simulink model "HvacCtrlrAirTFrnt"  (R2022b)
settings: Solver=FixedStepDiscrete  StartTime=0.0  StopTime=10.0  FixedStep=0.2  RelTol=1e-3
settings Model: Creator=blue.ge  LastModifiedBy=blue.ge
settings DataIOCC: SaveFormat=StructureWithTime
settings OptimizationCC: DefaultParameterBehavior=Inlined  EnhancedBackFolding=on  PassReuseOutputArgsAs=Individual arguments  ZeroExternalMemoryAtStartup=off  ZeroInternalMemoryAtStartup=off  OptimizeBlockOrder=speed  DifferentSizesBufferReuse=on  OptimizationCustomize=off  EfficientTunableParamExpr=on
settings DebuggingCC: ParameterTunabilityLossMsg=error
settings ModelReferenceCC: ModelReferenceNumInstancesAllowed=Single
settings SFSimCC: MATLABDynamicMemAlloc=off
settings RTWCC: Description=AUTOSAR  SystemTargetFile=autosar.tlc  GenCodeOnly=on  TemplateMakefile=ert_default_tmf
settings CodeAppCC: ForceParamTrailComments=on  IgnoreCustomStorageClasses=off  OperatorAnnotations=on  SimulinkDataObjDesc=on  SFDataObjDesc=on  InsertBlockDesc=on
settings STFCustomTargetCC: TargetLangStandard=C99 (ISO)  MultiwordLength=256  ParMdlRefBuildCompliant=on  CompOptLevelCompliant=on  IncludeMdlTerminateFcn=off  CombineOutputUpdateFcns=on  SuppressErrorStatus=on  ERTCustomFileBanners=on  SupportNonFinite=off  SupportComplex=off  SupportContinuousTime=off  SupportNonInlinedSFcns=off  RemoveResetFunc=on  MATLABClassNameForMDSCustomization=Simulink.SoftwareTarget.ERTCustomization  AutosarCompliant=on  UseToolchainInfoCompliant=on  LookupTableObjectStructAxisOrder=2,1,3,4,...  LUTObjectStructOrderEvenSpacing=Size,Table,Breakpoints  SystemTargetFile=autosar.tlc
settings ERTTargetCC: TargetLangStandard=C99 (ISO)  ConcurrentExecutionCompliant=off  IncludeMdlTerminateFcn=off  SuppressErrorStatus=on  SupportNonFinite=off  SupportComplex=off  ModelStepFunctionPrototypeControlCompliant=off  CPPClassGenCompliant=off  AutosarCompliant=on  LookupTableObjectStructAxisOrder=2,1,3,4,...  LUTObjectStructOrderEvenSpacing=Size,Table,Breakpoints  InlinedParameterPlacement=NonHierarchical  ERTCustomFileTemplate=example_file_process.tlc

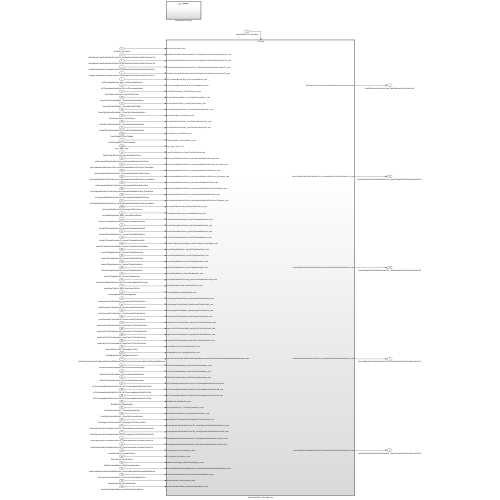
[thumbnail: Simulink's stored preview of the top-level diagram]
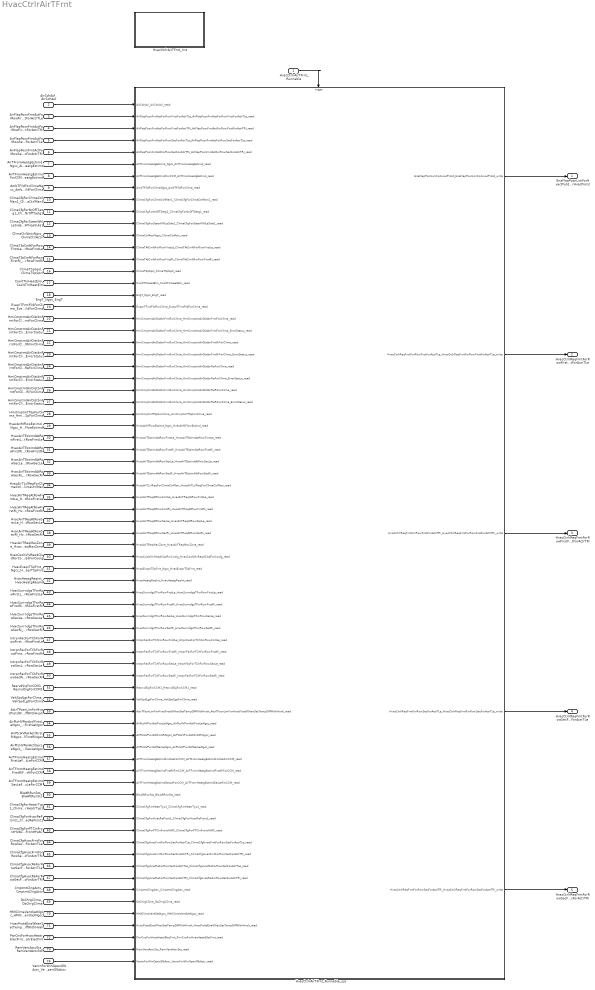
== system HvacCtrlrAirTFrnt ==
  HvacCtrlrAirTFrnt_Runnable : Inport  SampleTime=0.2  OutputFunctionCall=on
  AirCdnAvl_AirCdnAvl : Inport  Port=2  OutDataTypeStr=Boolean  PortDimensions=1
  AirFlapPosnFrntActForRowFirstForActrTLe_AirFlapPosnFrntActForRowFirstForActrTLe : Inport  Port=3  OutMin=0  OutMax=100  OutDataTypeStr=Perc9  PortDimensions=1
  AirFlapPosnFrntActForRowFirstForActrTRi_AirFlapPosnFrntActForRowFirstForActrTRi : Inport  Port=4  OutMin=0  OutMax=100  OutDataTypeStr=Perc9  PortDimensions=1
  AirFlapPosnFrntActForRowSecForActrTLe_AirFlapPosnFrntActForRowSecForActrTLe : Inport  Port=5  OutMin=0  OutMax=100  OutDataTypeStr=Perc9  PortDimensions=1
  AirFlapPosnFrntActForRowSecForActrTRi_AirFlapPosnFrntActForRowSecForActrTRi : Inport  Port=6  OutMin=0  OutMax=100  OutDataTypeStr=Perc9  PortDimensions=1
  AirTFromHeatgEstimd_Ngcc_AirTFromHeatgEstimd : Inport  Port=7  OutDataTypeStr=T30  PortDimensions=1
  AirTFromHeatgEstimdForCCM_AirTFromHeatgEstimd : Inport  Port=8  OutDataTypeStr=T30  PortDimensions=1
  AmbTFildForClimaNgcc_AmbTFildForClima : Inport  Port=9  OutDataTypeStr=T30  PortDimensions=1
  ClimaCfgForClimaCtrlMan1_ClimaCfgForClimaCtrlMan1 : Inport  Port=10  OutDataTypeStr=Boolean  PortDimensions=1
  ClimaCfgForNrOfTSetg1_ClimaCfgForNrOfTSetg1 : Inport  Port=11  OutDataTypeStr=Enum: ClimaCfgForNrOfTSetg1  PortDimensions=1
  ClimaCfgForSteerWhlLeSide1_ClimaCfgForSteerWhlLeSide1 : Inport  Port=12  OutDataTypeStr=Boolean  PortDimensions=1
  ClimaCtrlActvNgcc_ClimaCtrlActv : Inport  Port=13  OutDataTypeStr=Boolean  PortDimensions=1
  ClimaTAtCmftForRowFirstLe_ClimaTAtCmftForRowFirstLe : Inport  Port=14  OutDataTypeStr=T30  PortDimensions=1
  ClimaTAtCmftForRowFirstRi_ClimaTAtCmftForRowFirstRi : Inport  Port=15  OutDataTypeStr=T30  PortDimensions=1
  ClimaTSpSpcl_ClimaTSpSpcl : Inport  Port=16  OutDataTypeStr=Bus: ClimaTSpSpcl  PortDimensions=1  BusOutputAsStruct=on
  CooltTInHeatElm_CooltTInHeatElm : Inport  Port=17  OutDataTypeStr=T30  PortDimensions=1
  EngT_Ngcc_EngT : Inport  Port=18  OutDataTypeStr=Bus: EngT  PortDimensions=1  BusOutputAsStruct=on
  EvaprTFrntFildForClima_EvaprTFrntFildForClima : Inport  Port=19  OutDataTypeStr=T30  PortDimensions=1
  HmiCmptmtAirDistbnFrntForClima_HmiCmptmtAirDistbnFrntForClima : Inport  Port=20  OutDataTypeStr=Enum: HmiCmptmtAirDistbnFrnt  PortDimensions=1
  HmiCmptmtAirDistbnFrntForClima_HmiCmptmtAirDistbnFrntForClima_ErrorStatus : Inport  Port=21  OutDataTypeStr=uint8  PortDimensions=1
  HmiCmptmtAirDistbnFrntForClima_HmiCmptmtAirDistbnFrntRiForClima : Inport  Port=22  OutDataTypeStr=Enum: HmiCmptmtAirDistbnFrnt  PortDimensions=1
  HmiCmptmtAirDistbnFrntForClima_HmiCmptmtAirDistbnFrntRiForClima_ErrorStatus : Inport  Port=23  OutDataTypeStr=uint8  PortDimensions=1
  HmiCmptmtAirDistbnFrntForClima_HmiCmptmtAirDistbnReForClima : Inport  Port=24  OutDataTypeStr=Enum: HmiCmptmtAirDistbnFrnt  PortDimensions=1
  HmiCmptmtAirDistbnFrntForClima_HmiCmptmtAirDistbnReForClima_ErrorStatus : Inport  Port=25  OutDataTypeStr=uint8  PortDimensions=1
  HmiCmptmtAirDistbnFrntForClima_HmiCmptmtAirDistbnReRiForClima : Inport  Port=26  OutDataTypeStr=Enum: HmiCmptmtAirDistbnFrnt  PortDimensions=1
  HmiCmptmtAirDistbnFrntForClima_HmiCmptmtAirDistbnReRiForClima_ErrorStatus : Inport  Port=27  OutDataTypeStr=uint8  PortDimensions=1
  HmiCmptmtTSpForClima_HmiCmptmtTSpForClima : Inport  Port=28  OutDataTypeStr=Bus: HmiCmptmtTSp  PortDimensions=1  BusOutputAsStruct=on
  HvacAirMFlowEstimd_Ngcc_HvacAirMFlowEstimd : Inport  Port=29  OutMin=0  OutMax=1000  OutDataTypeStr=MFlow1  PortDimensions=1
  HvacAirTEstimdAtRowFirstLe_HvacAirTEstimdAtRowFirstLe : Inport  Port=30  OutDataTypeStr=T30  PortDimensions=1
  HvacAirTEstimdAtRowFirstRi_HvacAirTEstimdAtRowFirstRi : Inport  Port=31  OutDataTypeStr=T30  PortDimensions=1
  HvacAirTEstimdAtRowSecLe_HvacAirTEstimdAtRowSecLe : Inport  Port=32  OutDataTypeStr=T30  PortDimensions=1
  HvacAirTEstimdAtRowSecRi_HvacAirTEstimdAtRowSecRi : Inport  Port=33  OutDataTypeStr=T30  PortDimensions=1
  HvacAirTLvlReqForClimaCtrlMan_HvacAirTLvlReqForClimaCtrlMan : Inport  Port=34  OutDataTypeStr=Perc30  PortDimensions=1
  HvacAirTReqAtRowFirstLe_HvacAirTReqAtRowFirstLe : Inport  Port=35  OutDataTypeStr=T30  PortDimensions=1
  HvacAirTReqAtRowFirstRi_HvacAirTReqAtRowFirstRi : Inport  Port=36  OutDataTypeStr=T30  PortDimensions=1
  HvacAirTReqAtRowSecLe_HvacAirTReqAtRowSecLe : Inport  Port=37  OutDataTypeStr=T30  PortDimensions=1
  HvacAirTReqAtRowSecRi_HvacAirTReqAtRowSecRi : Inport  Port=38  OutDataTypeStr=T30  PortDimensions=1
  HvacAirTReqMaxZone_HvacAirTReqMaxZone : Inport  Port=39  OutDataTypeStr=Enum: TempZone  PortDimensions=1
  HvacCooltVlvReqdClsdForCoolg_HvacCooltVlvReqdClsdForCoolg : Inport  Port=40  OutDataTypeStr=Boolean  PortDimensions=1
  HvacEvaprTSpFrnt_Ngcc_HvacEvaprTSpFrnt : Inport  Port=41  OutDataTypeStr=HvacEvaprTSp  PortDimensions=1
  HvacHeatgReqInt_HvacHeatgReqInt : Inport  Port=42  OutDataTypeStr=Enum: OnOff1  PortDimensions=1
  HvacSurrndgsTForRowFirstLe_HvacSurrndgsTForRowFirstLe : Inport  Port=43  OutDataTypeStr=T30  PortDimensions=1
  HvacSurrndgsTForRowFirstRi_HvacSurrndgsTForRowFirstRi : Inport  Port=44  OutDataTypeStr=T30  PortDimensions=1
  HvacSurrndgsTForRowSecLe_HvacSurrndgsTForRowSecLe : Inport  Port=45  OutDataTypeStr=T30  PortDimensions=1
  HvacSurrndgsTForRowSecRi_HvacSurrndgsTForRowSecRi : Inport  Port=46  OutDataTypeStr=T30  PortDimensions=1
  IntrpnFacForTChForRowFirstLe_IntrpnFacForTChForRowFirstLe : Inport  Port=47  OutDataTypeStr=Fac30  PortDimensions=1
  IntrpnFacForTChForRowFirstRi_IntrpnFacForTChForRowFirstRi : Inport  Port=48  OutDataTypeStr=Fac30  PortDimensions=1
  IntrpnFacForTChForRowSecLe_IntrpnFacForTChForRowSecLe : Inport  Port=49  OutDataTypeStr=Fac30  PortDimensions=1
  IntrpnFacForTChForRowSecRi_IntrpnFacForTChForRowSecRi : Inport  Port=50  OutDataTypeStr=Fac30  PortDimensions=1
  ResrvdSigForCCM1_ResrvdSigForCCM1 : Inport  Port=51  OutDataTypeStr=BattIdTbl  PortDimensions=1
  VehSpdLgtForClima_VehSpdLgtForClima : Inport  Port=52  OutDataTypeStr=Bus: VehSpdLgtForClima  PortDimensions=1  BusOutputAsStruct=on
  ActrTPosnLimForHvacProtdWhenSetTempDiffWithHvah_ActrTPosnLimForHvacProtdWhenSetTempDiffWithHvah : Inport  Port=53  OutDataTypeStr=VCCPerc30  PortDimensions=1
  AirPtcHVPwrActlFirstLeNgcc_AirPtcHVPwrActlFirstLeNgcc : Inport  Port=54  OutMin=0  OutMax=20460  OutDataTypeStr=Pwr3  PortDimensions=1
  AirPtcHVPwrActlFirstRiNgcc_AirPtcHVPwrActlFirstRiNgcc : Inport  Port=55  OutMin=0  OutMax=20460  OutDataTypeStr=Pwr3  PortDimensions=1
  AirPtcHVPwrActlSecLeNgcc_AirPtcHVPwrActlSecLeNgcc : Inport  Port=56  OutMin=0  OutMax=20460  OutDataTypeStr=Pwr3  PortDimensions=1
  AirTFromHeatgEstimdFirstLeForCCM_AirTFromHeatgEstimdFirstLeForCCM : Inport  Port=57  OutDataTypeStr=T30  PortDimensions=1
  AirTFromHeatgEstimdFirstRiForCCM_AirTFromHeatgEstimdFirstRiForCCM : Inport  Port=58  OutDataTypeStr=T30  PortDimensions=1
  AirTFromHeatgEstimdSecLeForCCM_AirTFromHeatgEstimdSecLeForCCM : Inport  Port=59  OutDataTypeStr=T30  PortDimensions=1
  BlwAftRunSts_BlwAftRunSts : Inport  Port=60  OutDataTypeStr=Boolean  PortDimensions=1
  ClimaCfgForHeatrTyp1_ClimaCfgForHeatrTyp1 : Inport  Port=61  OutDataTypeStr=Enum: ClimaCfgForHeatrTyp1  PortDimensions=1
  ClimaCfgForHvacRePrsnt1_ClimaCfgForHvacRePrsnt1 : Inport  Port=62  OutDataTypeStr=Enum: CarCfgForHvacRe  PortDimensions=1
  ClimaCfgForPTCInFrontHVAC_ClimaCfgForPTCInFrontHVAC : Inport  Port=63  OutDataTypeStr=Enum: FrontHeaterExchanger  PortDimensions=1
  ClimaCfgHvacFrntForRowSecForActrTLe_ClimaCfgHvacFrntForRowSecForActrTLe : Inport  Port=64  OutDataTypeStr=Boolean  PortDimensions=1
  ClimaCfgHvacFrntForRowSecForActrTRi_ClimaCfgHvacFrntForRowSecForActrTRi : Inport  Port=65  OutDataTypeStr=Boolean  PortDimensions=1
  ClimaCfgHvacReForRowSecForActrTLe_ClimaCfgHvacReForRowSecForActrTLe : Inport  Port=66  OutDataTypeStr=UInt8  PortDimensions=1
  ClimaCfgHvacReForRowSecForActrTRi_ClimaCfgHvacReForRowSecForActrTRi : Inport  Port=67  OutDataTypeStr=UInt8  PortDimensions=1
  CmptmtClngActv_CmptmtClngActv : Inport  Port=68  OutDataTypeStr=Enum: CmptmtClngActv  PortDimensions=1
  DsChrgClima_DsChrgClima : Inport  Port=69  OutDataTypeStr=Boolean  PortDimensions=1
  HMIClimaVentSetNgcc_HMIClimaVentSetNgcc : Inport  Port=70  OutDataTypeStr=Enum: OnOff1  PortDimensions=1
  HvacProtdEnaWhenSetTempDiffWithHvah_HvacProtdEnaWhenSetTempDiffWithHvah : Inport  Port=71  OutDataTypeStr=Boolean  PortDimensions=1
  PwrCnsForHvacHeatrElecFrnt_PwrCnsForHvacHeatrElecFrnt : Inport  Port=72  OutDataTypeStr=Pwr33  PortDimensions=1
  RemVentActvSts_RemVentActvSts : Inport  Port=73  OutDataTypeStr=Enum: OnOff1  PortDimensions=1
  VentnForWinOpenGlbActv_VentnForWinOpenGlbActv : Inport  Port=74  OutDataTypeStr=Boolean  PortDimensions=1
  HvacCtrlrAirTFrnt_Init : Subsystem
  HvacCtrlrAirTFrnt_Runnable_sys : Subsystem
  EnaFlapPosnLimForHvacProtd_EnaFlapPosnLimForHvacProtd : Outport  OutDataTypeStr=Boolean  PortDimensions=1
  HvacCtrlrReqFrntForRowFirstForActrTLe_HvacCtrlrReqFrntForRowFirstForActrTLe : Outport  Port=2  OutDataTypeStr=Perc30  PortDimensions=1
  HvacCtrlrReqFrntForRowFirstForActrTRi_HvacCtrlrReqFrntForRowFirstForActrTRi : Outport  Port=3  OutDataTypeStr=Perc30  PortDimensions=1
  HvacCtrlrReqFrntForRowSecForActrTLe_HvacCtrlrReqFrntForRowSecForActrTLe : Outport  Port=4  OutDataTypeStr=Perc30  PortDimensions=1
  HvacCtrlrReqFrntForRowSecForActrTRi_HvacCtrlrReqFrntForRowSecForActrTRi : Outport  Port=5  OutDataTypeStr=Perc30  PortDimensions=1
  wires:
  AirCdnAvl_AirCdnAvl/1 -> HvacCtrlrAirTFrnt_Runnable_sys/1(AirCdnAvl_AirCdnAvl_read)
  AirFlapPosnFrntActForRowFirstForActrTLe_AirFlapPosnFrntActForRowFirstForActrTLe/1 -> HvacCtrlrAirTFrnt_Runnable_sys/2(AirFlapPosnFrntActForRowFirstForActrTLe_AirFlapPosnFrntActForRowFirstForActrTLe_read)
  AirFlapPosnFrntActForRowFirstForActrTRi_AirFlapPosnFrntActForRowFirstForActrTRi/1 -> HvacCtrlrAirTFrnt_Runnable_sys/3(AirFlapPosnFrntActForRowFirstForActrTRi_AirFlapPosnFrntActForRowFirstForActrTRi_read)
  AirFlapPosnFrntActForRowSecForActrTLe_AirFlapPosnFrntActForRowSecForActrTLe/1 -> HvacCtrlrAirTFrnt_Runnable_sys/4(AirFlapPosnFrntActForRowSecForActrTLe_AirFlapPosnFrntActForRowSecForActrTLe_read)
  AirFlapPosnFrntActForRowSecForActrTRi_AirFlapPosnFrntActForRowSecForActrTRi/1 -> HvacCtrlrAirTFrnt_Runnable_sys/5(AirFlapPosnFrntActForRowSecForActrTRi_AirFlapPosnFrntActForRowSecForActrTRi_read)
  AirTFromHeatgEstimd_Ngcc_AirTFromHeatgEstimd/1 -> HvacCtrlrAirTFrnt_Runnable_sys/6(AirTFromHeatgEstimd_Ngcc_AirTFromHeatgEstimd_read)
  AirTFromHeatgEstimdForCCM_AirTFromHeatgEstimd/1 -> HvacCtrlrAirTFrnt_Runnable_sys/7(AirTFromHeatgEstimdForCCM_AirTFromHeatgEstimd_read)
  AmbTFildForClimaNgcc_AmbTFildForClima/1 -> HvacCtrlrAirTFrnt_Runnable_sys/8(AmbTFildForClimaNgcc_AmbTFildForClima_read)
  ClimaCfgForClimaCtrlMan1_ClimaCfgForClimaCtrlMan1/1 -> HvacCtrlrAirTFrnt_Runnable_sys/9(ClimaCfgForClimaCtrlMan1_ClimaCfgForClimaCtrlMan1_read)
  ClimaCfgForNrOfTSetg1_ClimaCfgForNrOfTSetg1/1 -> HvacCtrlrAirTFrnt_Runnable_sys/10(ClimaCfgForNrOfTSetg1_ClimaCfgForNrOfTSetg1_read)
  ClimaCfgForSteerWhlLeSide1_ClimaCfgForSteerWhlLeSide1/1 -> HvacCtrlrAirTFrnt_Runnable_sys/11(ClimaCfgForSteerWhlLeSide1_ClimaCfgForSteerWhlLeSide1_read)
  ClimaCtrlActvNgcc_ClimaCtrlActv/1 -> HvacCtrlrAirTFrnt_Runnable_sys/12(ClimaCtrlActvNgcc_ClimaCtrlActv_read)
  ClimaTAtCmftForRowFirstLe_ClimaTAtCmftForRowFirstLe/1 -> HvacCtrlrAirTFrnt_Runnable_sys/13(ClimaTAtCmftForRowFirstLe_ClimaTAtCmftForRowFirstLe_read)
  ClimaTAtCmftForRowFirstRi_ClimaTAtCmftForRowFirstRi/1 -> HvacCtrlrAirTFrnt_Runnable_sys/14(ClimaTAtCmftForRowFirstRi_ClimaTAtCmftForRowFirstRi_read)
  ClimaTSpSpcl_ClimaTSpSpcl/1 -> HvacCtrlrAirTFrnt_Runnable_sys/15(ClimaTSpSpcl_ClimaTSpSpcl_read)
  CooltTInHeatElm_CooltTInHeatElm/1 -> HvacCtrlrAirTFrnt_Runnable_sys/16(CooltTInHeatElm_CooltTInHeatElm_read)
  EngT_Ngcc_EngT/1 -> HvacCtrlrAirTFrnt_Runnable_sys/17(EngT_Ngcc_EngT_read)
  EvaprTFrntFildForClima_EvaprTFrntFildForClima/1 -> HvacCtrlrAirTFrnt_Runnable_sys/18(EvaprTFrntFildForClima_EvaprTFrntFildForClima_read)
  HmiCmptmtAirDistbnFrntForClima_HmiCmptmtAirDistbnFrntForClima/1 -> HvacCtrlrAirTFrnt_Runnable_sys/19(HmiCmptmtAirDistbnFrntForClima_HmiCmptmtAirDistbnFrntForClima_read)
  HmiCmptmtAirDistbnFrntForClima_HmiCmptmtAirDistbnFrntForClima_ErrorStatus/1 -> HvacCtrlrAirTFrnt_Runnable_sys/20(HmiCmptmtAirDistbnFrntForClima_HmiCmptmtAirDistbnFrntForClima_ErrorStatus_read)
  HmiCmptmtAirDistbnFrntForClima_HmiCmptmtAirDistbnFrntRiForClima/1 -> HvacCtrlrAirTFrnt_Runnable_sys/21(HmiCmptmtAirDistbnFrntForClima_HmiCmptmtAirDistbnFrntRiForClima_read)
  HmiCmptmtAirDistbnFrntForClima_HmiCmptmtAirDistbnFrntRiForClima_ErrorStatus/1 -> HvacCtrlrAirTFrnt_Runnable_sys/22(HmiCmptmtAirDistbnFrntForClima_HmiCmptmtAirDistbnFrntRiForClima_ErrorStatus_read)
  HmiCmptmtAirDistbnFrntForClima_HmiCmptmtAirDistbnReForClima/1 -> HvacCtrlrAirTFrnt_Runnable_sys/23(HmiCmptmtAirDistbnFrntForClima_HmiCmptmtAirDistbnReForClima_read)
  HmiCmptmtAirDistbnFrntForClima_HmiCmptmtAirDistbnReForClima_ErrorStatus/1 -> HvacCtrlrAirTFrnt_Runnable_sys/24(HmiCmptmtAirDistbnFrntForClima_HmiCmptmtAirDistbnReForClima_ErrorStatus_read)
  HmiCmptmtAirDistbnFrntForClima_HmiCmptmtAirDistbnReRiForClima/1 -> HvacCtrlrAirTFrnt_Runnable_sys/25(HmiCmptmtAirDistbnFrntForClima_HmiCmptmtAirDistbnReRiForClima_read)
  HmiCmptmtAirDistbnFrntForClima_HmiCmptmtAirDistbnReRiForClima_ErrorStatus/1 -> HvacCtrlrAirTFrnt_Runnable_sys/26(HmiCmptmtAirDistbnFrntForClima_HmiCmptmtAirDistbnReRiForClima_ErrorStatus_read)
  HmiCmptmtTSpForClima_HmiCmptmtTSpForClima/1 -> HvacCtrlrAirTFrnt_Runnable_sys/27(HmiCmptmtTSpForClima_HmiCmptmtTSpForClima_read)
  HvacAirMFlowEstimd_Ngcc_HvacAirMFlowEstimd/1 -> HvacCtrlrAirTFrnt_Runnable_sys/28(HvacAirMFlowEstimd_Ngcc_HvacAirMFlowEstimd_read)
  HvacAirTEstimdAtRowFirstLe_HvacAirTEstimdAtRowFirstLe/1 -> HvacCtrlrAirTFrnt_Runnable_sys/29(HvacAirTEstimdAtRowFirstLe_HvacAirTEstimdAtRowFirstLe_read)
  HvacAirTEstimdAtRowFirstRi_HvacAirTEstimdAtRowFirstRi/1 -> HvacCtrlrAirTFrnt_Runnable_sys/30(HvacAirTEstimdAtRowFirstRi_HvacAirTEstimdAtRowFirstRi_read)
  HvacAirTEstimdAtRowSecLe_HvacAirTEstimdAtRowSecLe/1 -> HvacCtrlrAirTFrnt_Runnable_sys/31(HvacAirTEstimdAtRowSecLe_HvacAirTEstimdAtRowSecLe_read)
  HvacAirTEstimdAtRowSecRi_HvacAirTEstimdAtRowSecRi/1 -> HvacCtrlrAirTFrnt_Runnable_sys/32(HvacAirTEstimdAtRowSecRi_HvacAirTEstimdAtRowSecRi_read)
  HvacAirTLvlReqForClimaCtrlMan_HvacAirTLvlReqForClimaCtrlMan/1 -> HvacCtrlrAirTFrnt_Runnable_sys/33(HvacAirTLvlReqForClimaCtrlMan_HvacAirTLvlReqForClimaCtrlMan_read)
  HvacAirTReqAtRowFirstLe_HvacAirTReqAtRowFirstLe/1 -> HvacCtrlrAirTFrnt_Runnable_sys/34(HvacAirTReqAtRowFirstLe_HvacAirTReqAtRowFirstLe_read)
  HvacAirTReqAtRowFirstRi_HvacAirTReqAtRowFirstRi/1 -> HvacCtrlrAirTFrnt_Runnable_sys/35(HvacAirTReqAtRowFirstRi_HvacAirTReqAtRowFirstRi_read)
  HvacAirTReqAtRowSecLe_HvacAirTReqAtRowSecLe/1 -> HvacCtrlrAirTFrnt_Runnable_sys/36(HvacAirTReqAtRowSecLe_HvacAirTReqAtRowSecLe_read)
  HvacAirTReqAtRowSecRi_HvacAirTReqAtRowSecRi/1 -> HvacCtrlrAirTFrnt_Runnable_sys/37(HvacAirTReqAtRowSecRi_HvacAirTReqAtRowSecRi_read)
  HvacAirTReqMaxZone_HvacAirTReqMaxZone/1 -> HvacCtrlrAirTFrnt_Runnable_sys/38(HvacAirTReqMaxZone_HvacAirTReqMaxZone_read)
  HvacCooltVlvReqdClsdForCoolg_HvacCooltVlvReqdClsdForCoolg/1 -> HvacCtrlrAirTFrnt_Runnable_sys/39(HvacCooltVlvReqdClsdForCoolg_HvacCooltVlvReqdClsdForCoolg_read)
  HvacEvaprTSpFrnt_Ngcc_HvacEvaprTSpFrnt/1 -> HvacCtrlrAirTFrnt_Runnable_sys/40(HvacEvaprTSpFrnt_Ngcc_HvacEvaprTSpFrnt_read)
  HvacHeatgReqInt_HvacHeatgReqInt/1 -> HvacCtrlrAirTFrnt_Runnable_sys/41(HvacHeatgReqInt_HvacHeatgReqInt_read)
  HvacSurrndgsTForRowFirstLe_HvacSurrndgsTForRowFirstLe/1 -> HvacCtrlrAirTFrnt_Runnable_sys/42(HvacSurrndgsTForRowFirstLe_HvacSurrndgsTForRowFirstLe_read)
  HvacSurrndgsTForRowFirstRi_HvacSurrndgsTForRowFirstRi/1 -> HvacCtrlrAirTFrnt_Runnable_sys/43(HvacSurrndgsTForRowFirstRi_HvacSurrndgsTForRowFirstRi_read)
  HvacSurrndgsTForRowSecLe_HvacSurrndgsTForRowSecLe/1 -> HvacCtrlrAirTFrnt_Runnable_sys/44(HvacSurrndgsTForRowSecLe_HvacSurrndgsTForRowSecLe_read)
  HvacSurrndgsTForRowSecRi_HvacSurrndgsTForRowSecRi/1 -> HvacCtrlrAirTFrnt_Runnable_sys/45(HvacSurrndgsTForRowSecRi_HvacSurrndgsTForRowSecRi_read)
  IntrpnFacForTChForRowFirstLe_IntrpnFacForTChForRowFirstLe/1 -> HvacCtrlrAirTFrnt_Runnable_sys/46(IntrpnFacForTChForRowFirstLe_IntrpnFacForTChForRowFirstLe_read)
  IntrpnFacForTChForRowFirstRi_IntrpnFacForTChForRowFirstRi/1 -> HvacCtrlrAirTFrnt_Runnable_sys/47(IntrpnFacForTChForRowFirstRi_IntrpnFacForTChForRowFirstRi_read)
  IntrpnFacForTChForRowSecLe_IntrpnFacForTChForRowSecLe/1 -> HvacCtrlrAirTFrnt_Runnable_sys/48(IntrpnFacForTChForRowSecLe_IntrpnFacForTChForRowSecLe_read)
  IntrpnFacForTChForRowSecRi_IntrpnFacForTChForRowSecRi/1 -> HvacCtrlrAirTFrnt_Runnable_sys/49(IntrpnFacForTChForRowSecRi_IntrpnFacForTChForRowSecRi_read)
  ResrvdSigForCCM1_ResrvdSigForCCM1/1 -> HvacCtrlrAirTFrnt_Runnable_sys/50(ResrvdSigForCCM1_ResrvdSigForCCM1_read)
  VehSpdLgtForClima_VehSpdLgtForClima/1 -> HvacCtrlrAirTFrnt_Runnable_sys/51(VehSpdLgtForClima_VehSpdLgtForClima_read)
  ActrTPosnLimForHvacProtdWhenSetTempDiffWithHvah_ActrTPosnLimForHvacProtdWhenSetTempDiffWithHvah/1 -> HvacCtrlrAirTFrnt_Runnable_sys/52(ActrTPosnLimForHvacProtdWhenSetTempDiffWithHvah_ActrTPosnLimForHvacProtdWhenSetTempDiffWithHvah_read)
  AirPtcHVPwrActlFirstLeNgcc_AirPtcHVPwrActlFirstLeNgcc/1 -> HvacCtrlrAirTFrnt_Runnable_sys/53(AirPtcHVPwrActlFirstLeNgcc_AirPtcHVPwrActlFirstLeNgcc_read)
  AirPtcHVPwrActlFirstRiNgcc_AirPtcHVPwrActlFirstRiNgcc/1 -> HvacCtrlrAirTFrnt_Runnable_sys/54(AirPtcHVPwrActlFirstRiNgcc_AirPtcHVPwrActlFirstRiNgcc_read)
  AirPtcHVPwrActlSecLeNgcc_AirPtcHVPwrActlSecLeNgcc/1 -> HvacCtrlrAirTFrnt_Runnable_sys/55(AirPtcHVPwrActlSecLeNgcc_AirPtcHVPwrActlSecLeNgcc_read)
  AirTFromHeatgEstimdFirstLeForCCM_AirTFromHeatgEstimdFirstLeForCCM/1 -> HvacCtrlrAirTFrnt_Runnable_sys/56(AirTFromHeatgEstimdFirstLeForCCM_AirTFromHeatgEstimdFirstLeForCCM_read)
  AirTFromHeatgEstimdFirstRiForCCM_AirTFromHeatgEstimdFirstRiForCCM/1 -> HvacCtrlrAirTFrnt_Runnable_sys/57(AirTFromHeatgEstimdFirstRiForCCM_AirTFromHeatgEstimdFirstRiForCCM_read)
  AirTFromHeatgEstimdSecLeForCCM_AirTFromHeatgEstimdSecLeForCCM/1 -> HvacCtrlrAirTFrnt_Runnable_sys/58(AirTFromHeatgEstimdSecLeForCCM_AirTFromHeatgEstimdSecLeForCCM_read)
  BlwAftRunSts_BlwAftRunSts/1 -> HvacCtrlrAirTFrnt_Runnable_sys/59(BlwAftRunSts_BlwAftRunSts_read)
  ClimaCfgForHeatrTyp1_ClimaCfgForHeatrTyp1/1 -> HvacCtrlrAirTFrnt_Runnable_sys/60(ClimaCfgForHeatrTyp1_ClimaCfgForHeatrTyp1_read)
  ClimaCfgForHvacRePrsnt1_ClimaCfgForHvacRePrsnt1/1 -> HvacCtrlrAirTFrnt_Runnable_sys/61(ClimaCfgForHvacRePrsnt1_ClimaCfgForHvacRePrsnt1_read)
  ClimaCfgForPTCInFrontHVAC_ClimaCfgForPTCInFrontHVAC/1 -> HvacCtrlrAirTFrnt_Runnable_sys/62(ClimaCfgForPTCInFrontHVAC_ClimaCfgForPTCInFrontHVAC_read)
  ClimaCfgHvacFrntForRowSecForActrTLe_ClimaCfgHvacFrntForRowSecForActrTLe/1 -> HvacCtrlrAirTFrnt_Runnable_sys/63(ClimaCfgHvacFrntForRowSecForActrTLe_ClimaCfgHvacFrntForRowSecForActrTLe_read)
  ClimaCfgHvacFrntForRowSecForActrTRi_ClimaCfgHvacFrntForRowSecForActrTRi/1 -> HvacCtrlrAirTFrnt_Runnable_sys/64(ClimaCfgHvacFrntForRowSecForActrTRi_ClimaCfgHvacFrntForRowSecForActrTRi_read)
  ClimaCfgHvacReForRowSecForActrTLe_ClimaCfgHvacReForRowSecForActrTLe/1 -> HvacCtrlrAirTFrnt_Runnable_sys/65(ClimaCfgHvacReForRowSecForActrTLe_ClimaCfgHvacReForRowSecForActrTLe_read)
  ClimaCfgHvacReForRowSecForActrTRi_ClimaCfgHvacReForRowSecForActrTRi/1 -> HvacCtrlrAirTFrnt_Runnable_sys/66(ClimaCfgHvacReForRowSecForActrTRi_ClimaCfgHvacReForRowSecForActrTRi_read)
  CmptmtClngActv_CmptmtClngActv/1 -> HvacCtrlrAirTFrnt_Runnable_sys/67(CmptmtClngActv_CmptmtClngActv_read)
  DsChrgClima_DsChrgClima/1 -> HvacCtrlrAirTFrnt_Runnable_sys/68(DsChrgClima_DsChrgClima_read)
  HMIClimaVentSetNgcc_HMIClimaVentSetNgcc/1 -> HvacCtrlrAirTFrnt_Runnable_sys/69(HMIClimaVentSetNgcc_HMIClimaVentSetNgcc_read)
  HvacProtdEnaWhenSetTempDiffWithHvah_HvacProtdEnaWhenSetTempDiffWithHvah/1 -> HvacCtrlrAirTFrnt_Runnable_sys/70(HvacProtdEnaWhenSetTempDiffWithHvah_HvacProtdEnaWhenSetTempDiffWithHvah_read)
  PwrCnsForHvacHeatrElecFrnt_PwrCnsForHvacHeatrElecFrnt/1 -> HvacCtrlrAirTFrnt_Runnable_sys/71(PwrCnsForHvacHeatrElecFrnt_PwrCnsForHvacHeatrElecFrnt_read)
  RemVentActvSts_RemVentActvSts/1 -> HvacCtrlrAirTFrnt_Runnable_sys/72(RemVentActvSts_RemVentActvSts_read)
  VentnForWinOpenGlbActv_VentnForWinOpenGlbActv/1 -> HvacCtrlrAirTFrnt_Runnable_sys/73(VentnForWinOpenGlbActv_VentnForWinOpenGlbActv_read)
  HvacCtrlrAirTFrnt_Runnable_sys/1(EnaFlapPosnLimForHvacProtd_EnaFlapPosnLimForHvacProtd_write) -> EnaFlapPosnLimForHvacProtd_EnaFlapPosnLimForHvacProtd/1
  HvacCtrlrAirTFrnt_Runnable_sys/2(HvacCtrlrReqFrntForRowFirstForActrTLe_HvacCtrlrReqFrntForRowFirstForActrTLe_write) -> HvacCtrlrReqFrntForRowFirstForActrTLe_HvacCtrlrReqFrntForRowFirstForActrTLe/1
  HvacCtrlrAirTFrnt_Runnable_sys/3(HvacCtrlrReqFrntForRowFirstForActrTRi_HvacCtrlrReqFrntForRowFirstForActrTRi_write) -> HvacCtrlrReqFrntForRowFirstForActrTRi_HvacCtrlrReqFrntForRowFirstForActrTRi/1
  HvacCtrlrAirTFrnt_Runnable_sys/4(HvacCtrlrReqFrntForRowSecForActrTLe_HvacCtrlrReqFrntForRowSecForActrTLe_write) -> HvacCtrlrReqFrntForRowSecForActrTLe_HvacCtrlrReqFrntForRowSecForActrTLe/1
  HvacCtrlrAirTFrnt_Runnable_sys/5(HvacCtrlrReqFrntForRowSecForActrTRi_HvacCtrlrReqFrntForRowSecForActrTRi_write) -> HvacCtrlrReqFrntForRowSecForActrTRi_HvacCtrlrReqFrntForRowSecForActrTRi/1
  HvacCtrlrAirTFrnt_Runnable/1 -> HvacCtrlrAirTFrnt_Runnable_sys/Trigger

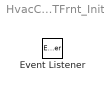
== system HvacCtrlrAirTFrnt/HvacCtrlrAirTFrnt_Init ==
  Event Listener : EventListener  EventName=reset

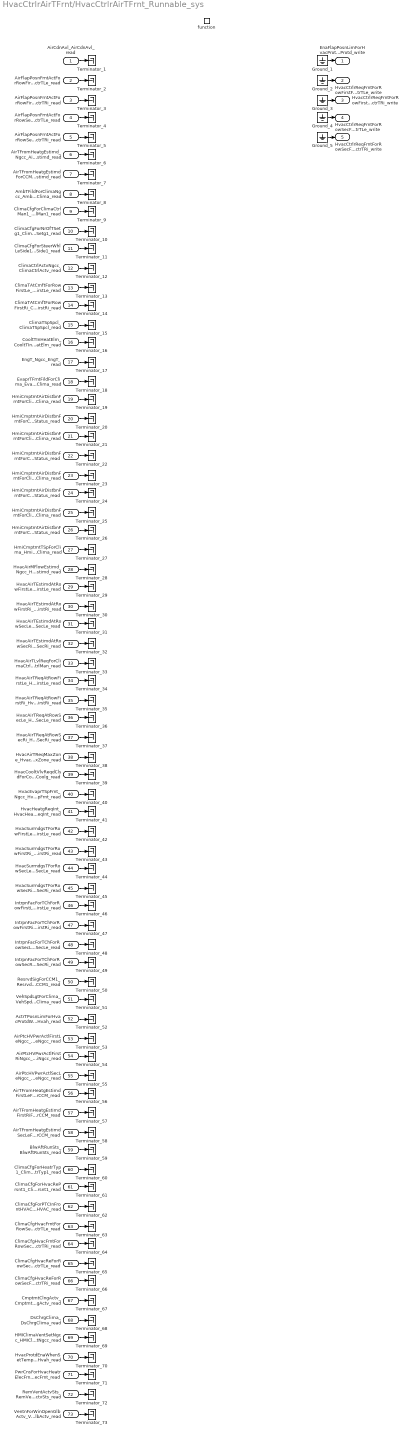
== system HvacCtrlrAirTFrnt/HvacCtrlrAirTFrnt_Runnable_sys ==
  AirCdnAvl_AirCdnAvl_read : Inport
  AirFlapPosnFrntActForRowFirstForActrTLe_AirFlapPosnFrntActForRowFirstForActrTLe_read : Inport  Port=2
  AirFlapPosnFrntActForRowFirstForActrTRi_AirFlapPosnFrntActForRowFirstForActrTRi_read : Inport  Port=3
  AirFlapPosnFrntActForRowSecForActrTLe_AirFlapPosnFrntActForRowSecForActrTLe_read : Inport  Port=4
  AirFlapPosnFrntActForRowSecForActrTRi_AirFlapPosnFrntActForRowSecForActrTRi_read : Inport  Port=5
  AirTFromHeatgEstimd_Ngcc_AirTFromHeatgEstimd_read : Inport  Port=6
  AirTFromHeatgEstimdForCCM_AirTFromHeatgEstimd_read : Inport  Port=7
  AmbTFildForClimaNgcc_AmbTFildForClima_read : Inport  Port=8
  ClimaCfgForClimaCtrlMan1_ClimaCfgForClimaCtrlMan1_read : Inport  Port=9
  ClimaCfgForNrOfTSetg1_ClimaCfgForNrOfTSetg1_read : Inport  Port=10
  ClimaCfgForSteerWhlLeSide1_ClimaCfgForSteerWhlLeSide1_read : Inport  Port=11
  ClimaCtrlActvNgcc_ClimaCtrlActv_read : Inport  Port=12
  ClimaTAtCmftForRowFirstLe_ClimaTAtCmftForRowFirstLe_read : Inport  Port=13
  ClimaTAtCmftForRowFirstRi_ClimaTAtCmftForRowFirstRi_read : Inport  Port=14
  ClimaTSpSpcl_ClimaTSpSpcl_read : Inport  Port=15
  CooltTInHeatElm_CooltTInHeatElm_read : Inport  Port=16
  EngT_Ngcc_EngT_read : Inport  Port=17
  EvaprTFrntFildForClima_EvaprTFrntFildForClima_read : Inport  Port=18
  HmiCmptmtAirDistbnFrntForClima_HmiCmptmtAirDistbnFrntForClima_read : Inport  Port=19
  HmiCmptmtAirDistbnFrntForClima_HmiCmptmtAirDistbnFrntForClima_ErrorStatus_read : Inport  Port=20
  HmiCmptmtAirDistbnFrntForClima_HmiCmptmtAirDistbnFrntRiForClima_read : Inport  Port=21
  HmiCmptmtAirDistbnFrntForClima_HmiCmptmtAirDistbnFrntRiForClima_ErrorStatus_read : Inport  Port=22
  HmiCmptmtAirDistbnFrntForClima_HmiCmptmtAirDistbnReForClima_read : Inport  Port=23
  HmiCmptmtAirDistbnFrntForClima_HmiCmptmtAirDistbnReForClima_ErrorStatus_read : Inport  Port=24
  HmiCmptmtAirDistbnFrntForClima_HmiCmptmtAirDistbnReRiForClima_read : Inport  Port=25
  HmiCmptmtAirDistbnFrntForClima_HmiCmptmtAirDistbnReRiForClima_ErrorStatus_read : Inport  Port=26
  HmiCmptmtTSpForClima_HmiCmptmtTSpForClima_read : Inport  Port=27
  HvacAirMFlowEstimd_Ngcc_HvacAirMFlowEstimd_read : Inport  Port=28
  HvacAirTEstimdAtRowFirstLe_HvacAirTEstimdAtRowFirstLe_read : Inport  Port=29
  HvacAirTEstimdAtRowFirstRi_HvacAirTEstimdAtRowFirstRi_read : Inport  Port=30
  HvacAirTEstimdAtRowSecLe_HvacAirTEstimdAtRowSecLe_read : Inport  Port=31
  HvacAirTEstimdAtRowSecRi_HvacAirTEstimdAtRowSecRi_read : Inport  Port=32
  HvacAirTLvlReqForClimaCtrlMan_HvacAirTLvlReqForClimaCtrlMan_read : Inport  Port=33
  HvacAirTReqAtRowFirstLe_HvacAirTReqAtRowFirstLe_read : Inport  Port=34
  HvacAirTReqAtRowFirstRi_HvacAirTReqAtRowFirstRi_read : Inport  Port=35
  HvacAirTReqAtRowSecLe_HvacAirTReqAtRowSecLe_read : Inport  Port=36
  HvacAirTReqAtRowSecRi_HvacAirTReqAtRowSecRi_read : Inport  Port=37
  HvacAirTReqMaxZone_HvacAirTReqMaxZone_read : Inport  Port=38
  HvacCooltVlvReqdClsdForCoolg_HvacCooltVlvReqdClsdForCoolg_read : Inport  Port=39
  HvacEvaprTSpFrnt_Ngcc_HvacEvaprTSpFrnt_read : Inport  Port=40
  HvacHeatgReqInt_HvacHeatgReqInt_read : Inport  Port=41
  HvacSurrndgsTForRowFirstLe_HvacSurrndgsTForRowFirstLe_read : Inport  Port=42
  HvacSurrndgsTForRowFirstRi_HvacSurrndgsTForRowFirstRi_read : Inport  Port=43
  HvacSurrndgsTForRowSecLe_HvacSurrndgsTForRowSecLe_read : Inport  Port=44
  HvacSurrndgsTForRowSecRi_HvacSurrndgsTForRowSecRi_read : Inport  Port=45
  IntrpnFacForTChForRowFirstLe_IntrpnFacForTChForRowFirstLe_read : Inport  Port=46
  IntrpnFacForTChForRowFirstRi_IntrpnFacForTChForRowFirstRi_read : Inport  Port=47
  IntrpnFacForTChForRowSecLe_IntrpnFacForTChForRowSecLe_read : Inport  Port=48
  IntrpnFacForTChForRowSecRi_IntrpnFacForTChForRowSecRi_read : Inport  Port=49
  ResrvdSigForCCM1_ResrvdSigForCCM1_read : Inport  Port=50
  VehSpdLgtForClima_VehSpdLgtForClima_read : Inport  Port=51
  ActrTPosnLimForHvacProtdWhenSetTempDiffWithHvah_ActrTPosnLimForHvacProtdWhenSetTempDiffWithHvah_read : Inport  Port=52
  AirPtcHVPwrActlFirstLeNgcc_AirPtcHVPwrActlFirstLeNgcc_read : Inport  Port=53
  AirPtcHVPwrActlFirstRiNgcc_AirPtcHVPwrActlFirstRiNgcc_read : Inport  Port=54
  AirPtcHVPwrActlSecLeNgcc_AirPtcHVPwrActlSecLeNgcc_read : Inport  Port=55
  AirTFromHeatgEstimdFirstLeForCCM_AirTFromHeatgEstimdFirstLeForCCM_read : Inport  Port=56
  AirTFromHeatgEstimdFirstRiForCCM_AirTFromHeatgEstimdFirstRiForCCM_read : Inport  Port=57
  AirTFromHeatgEstimdSecLeForCCM_AirTFromHeatgEstimdSecLeForCCM_read : Inport  Port=58
  BlwAftRunSts_BlwAftRunSts_read : Inport  Port=59
  ClimaCfgForHeatrTyp1_ClimaCfgForHeatrTyp1_read : Inport  Port=60
  ClimaCfgForHvacRePrsnt1_ClimaCfgForHvacRePrsnt1_read : Inport  Port=61
  ClimaCfgForPTCInFrontHVAC_ClimaCfgForPTCInFrontHVAC_read : Inport  Port=62
  ClimaCfgHvacFrntForRowSecForActrTLe_ClimaCfgHvacFrntForRowSecForActrTLe_read : Inport  Port=63
  ClimaCfgHvacFrntForRowSecForActrTRi_ClimaCfgHvacFrntForRowSecForActrTRi_read : Inport  Port=64
  ClimaCfgHvacReForRowSecForActrTLe_ClimaCfgHvacReForRowSecForActrTLe_read : Inport  Port=65
  ClimaCfgHvacReForRowSecForActrTRi_ClimaCfgHvacReForRowSecForActrTRi_read : Inport  Port=66
  CmptmtClngActv_CmptmtClngActv_read : Inport  Port=67
  DsChrgClima_DsChrgClima_read : Inport  Port=68
  HMIClimaVentSetNgcc_HMIClimaVentSetNgcc_read : Inport  Port=69
  HvacProtdEnaWhenSetTempDiffWithHvah_HvacProtdEnaWhenSetTempDiffWithHvah_read : Inport  Port=70
  PwrCnsForHvacHeatrElecFrnt_PwrCnsForHvacHeatrElecFrnt_read : Inport  Port=71
  RemVentActvSts_RemVentActvSts_read : Inport  Port=72
  VentnForWinOpenGlbActv_VentnForWinOpenGlbActv_read : Inport  Port=73
  function : TriggerPort  TriggerType=function-call  VariantControl=(inherit)  SampleTimeType=periodic  SampleTime=-1
  Ground_{1..5} (5 alike) : Ground
  Terminator_{1..73} (73 alike) : Terminator
  EnaFlapPosnLimForHvacProtd_EnaFlapPosnLimForHvacProtd_write : Outport
      settings: EnsureOutportIsVirtual=on
  HvacCtrlrReqFrntForRowFirstForActrTLe_HvacCtrlrReqFrntForRowFirstForActrTLe_write : Outport  Port=2
      settings: EnsureOutportIsVirtual=on
  HvacCtrlrReqFrntForRowFirstForActrTRi_HvacCtrlrReqFrntForRowFirstForActrTRi_write : Outport  Port=3
      settings: EnsureOutportIsVirtual=on
  HvacCtrlrReqFrntForRowSecForActrTLe_HvacCtrlrReqFrntForRowSecForActrTLe_write : Outport  Port=4
      settings: EnsureOutportIsVirtual=on
  HvacCtrlrReqFrntForRowSecForActrTRi_HvacCtrlrReqFrntForRowSecForActrTRi_write : Outport  Port=5
      settings: EnsureOutportIsVirtual=on
  wires:
  AirCdnAvl_AirCdnAvl_read/1 -> Terminator_1/1
  AirFlapPosnFrntActForRowFirstForActrTLe_AirFlapPosnFrntActForRowFirstForActrTLe_read/1 -> Terminator_2/1
  AirFlapPosnFrntActForRowFirstForActrTRi_AirFlapPosnFrntActForRowFirstForActrTRi_read/1 -> Terminator_3/1
  AirFlapPosnFrntActForRowSecForActrTLe_AirFlapPosnFrntActForRowSecForActrTLe_read/1 -> Terminator_4/1
  AirFlapPosnFrntActForRowSecForActrTRi_AirFlapPosnFrntActForRowSecForActrTRi_read/1 -> Terminator_5/1
  AirTFromHeatgEstimd_Ngcc_AirTFromHeatgEstimd_read/1 -> Terminator_6/1
  AirTFromHeatgEstimdForCCM_AirTFromHeatgEstimd_read/1 -> Terminator_7/1
  AmbTFildForClimaNgcc_AmbTFildForClima_read/1 -> Terminator_8/1
  ClimaCfgForClimaCtrlMan1_ClimaCfgForClimaCtrlMan1_read/1 -> Terminator_9/1
  ClimaCfgForNrOfTSetg1_ClimaCfgForNrOfTSetg1_read/1 -> Terminator_10/1
  ClimaCfgForSteerWhlLeSide1_ClimaCfgForSteerWhlLeSide1_read/1 -> Terminator_11/1
  ClimaCtrlActvNgcc_ClimaCtrlActv_read/1 -> Terminator_12/1
  ClimaTAtCmftForRowFirstLe_ClimaTAtCmftForRowFirstLe_read/1 -> Terminator_13/1
  ClimaTAtCmftForRowFirstRi_ClimaTAtCmftForRowFirstRi_read/1 -> Terminator_14/1
  ClimaTSpSpcl_ClimaTSpSpcl_read/1 -> Terminator_15/1
  CooltTInHeatElm_CooltTInHeatElm_read/1 -> Terminator_16/1
  EngT_Ngcc_EngT_read/1 -> Terminator_17/1
  EvaprTFrntFildForClima_EvaprTFrntFildForClima_read/1 -> Terminator_18/1
  HmiCmptmtAirDistbnFrntForClima_HmiCmptmtAirDistbnFrntForClima_read/1 -> Terminator_19/1
  HmiCmptmtAirDistbnFrntForClima_HmiCmptmtAirDistbnFrntForClima_ErrorStatus_read/1 -> Terminator_20/1
  HmiCmptmtAirDistbnFrntForClima_HmiCmptmtAirDistbnFrntRiForClima_read/1 -> Terminator_21/1
  HmiCmptmtAirDistbnFrntForClima_HmiCmptmtAirDistbnFrntRiForClima_ErrorStatus_read/1 -> Terminator_22/1
  HmiCmptmtAirDistbnFrntForClima_HmiCmptmtAirDistbnReForClima_read/1 -> Terminator_23/1
  HmiCmptmtAirDistbnFrntForClima_HmiCmptmtAirDistbnReForClima_ErrorStatus_read/1 -> Terminator_24/1
  HmiCmptmtAirDistbnFrntForClima_HmiCmptmtAirDistbnReRiForClima_read/1 -> Terminator_25/1
  HmiCmptmtAirDistbnFrntForClima_HmiCmptmtAirDistbnReRiForClima_ErrorStatus_read/1 -> Terminator_26/1
  HmiCmptmtTSpForClima_HmiCmptmtTSpForClima_read/1 -> Terminator_27/1
  HvacAirMFlowEstimd_Ngcc_HvacAirMFlowEstimd_read/1 -> Terminator_28/1
  HvacAirTEstimdAtRowFirstLe_HvacAirTEstimdAtRowFirstLe_read/1 -> Terminator_29/1
  HvacAirTEstimdAtRowFirstRi_HvacAirTEstimdAtRowFirstRi_read/1 -> Terminator_30/1
  HvacAirTEstimdAtRowSecLe_HvacAirTEstimdAtRowSecLe_read/1 -> Terminator_31/1
  HvacAirTEstimdAtRowSecRi_HvacAirTEstimdAtRowSecRi_read/1 -> Terminator_32/1
  HvacAirTLvlReqForClimaCtrlMan_HvacAirTLvlReqForClimaCtrlMan_read/1 -> Terminator_33/1
  HvacAirTReqAtRowFirstLe_HvacAirTReqAtRowFirstLe_read/1 -> Terminator_34/1
  HvacAirTReqAtRowFirstRi_HvacAirTReqAtRowFirstRi_read/1 -> Terminator_35/1
  HvacAirTReqAtRowSecLe_HvacAirTReqAtRowSecLe_read/1 -> Terminator_36/1
  HvacAirTReqAtRowSecRi_HvacAirTReqAtRowSecRi_read/1 -> Terminator_37/1
  HvacAirTReqMaxZone_HvacAirTReqMaxZone_read/1 -> Terminator_38/1
  HvacCooltVlvReqdClsdForCoolg_HvacCooltVlvReqdClsdForCoolg_read/1 -> Terminator_39/1
  HvacEvaprTSpFrnt_Ngcc_HvacEvaprTSpFrnt_read/1 -> Terminator_40/1
  HvacHeatgReqInt_HvacHeatgReqInt_read/1 -> Terminator_41/1
  HvacSurrndgsTForRowFirstLe_HvacSurrndgsTForRowFirstLe_read/1 -> Terminator_42/1
  HvacSurrndgsTForRowFirstRi_HvacSurrndgsTForRowFirstRi_read/1 -> Terminator_43/1
  HvacSurrndgsTForRowSecLe_HvacSurrndgsTForRowSecLe_read/1 -> Terminator_44/1
  HvacSurrndgsTForRowSecRi_HvacSurrndgsTForRowSecRi_read/1 -> Terminator_45/1
  IntrpnFacForTChForRowFirstLe_IntrpnFacForTChForRowFirstLe_read/1 -> Terminator_46/1
  IntrpnFacForTChForRowFirstRi_IntrpnFacForTChForRowFirstRi_read/1 -> Terminator_47/1
  IntrpnFacForTChForRowSecLe_IntrpnFacForTChForRowSecLe_read/1 -> Terminator_48/1
  IntrpnFacForTChForRowSecRi_IntrpnFacForTChForRowSecRi_read/1 -> Terminator_49/1
  ResrvdSigForCCM1_ResrvdSigForCCM1_read/1 -> Terminator_50/1
  VehSpdLgtForClima_VehSpdLgtForClima_read/1 -> Terminator_51/1
  ActrTPosnLimForHvacProtdWhenSetTempDiffWithHvah_ActrTPosnLimForHvacProtdWhenSetTempDiffWithHvah_read/1 -> Terminator_52/1
  AirPtcHVPwrActlFirstLeNgcc_AirPtcHVPwrActlFirstLeNgcc_read/1 -> Terminator_53/1
  AirPtcHVPwrActlFirstRiNgcc_AirPtcHVPwrActlFirstRiNgcc_read/1 -> Terminator_54/1
  AirPtcHVPwrActlSecLeNgcc_AirPtcHVPwrActlSecLeNgcc_read/1 -> Terminator_55/1
  AirTFromHeatgEstimdFirstLeForCCM_AirTFromHeatgEstimdFirstLeForCCM_read/1 -> Terminator_56/1
  AirTFromHeatgEstimdFirstRiForCCM_AirTFromHeatgEstimdFirstRiForCCM_read/1 -> Terminator_57/1
  AirTFromHeatgEstimdSecLeForCCM_AirTFromHeatgEstimdSecLeForCCM_read/1 -> Terminator_58/1
  BlwAftRunSts_BlwAftRunSts_read/1 -> Terminator_59/1
  ClimaCfgForHeatrTyp1_ClimaCfgForHeatrTyp1_read/1 -> Terminator_60/1
  ClimaCfgForHvacRePrsnt1_ClimaCfgForHvacRePrsnt1_read/1 -> Terminator_61/1
  ClimaCfgForPTCInFrontHVAC_ClimaCfgForPTCInFrontHVAC_read/1 -> Terminator_62/1
  ClimaCfgHvacFrntForRowSecForActrTLe_ClimaCfgHvacFrntForRowSecForActrTLe_read/1 -> Terminator_63/1
  ClimaCfgHvacFrntForRowSecForActrTRi_ClimaCfgHvacFrntForRowSecForActrTRi_read/1 -> Terminator_64/1
  ClimaCfgHvacReForRowSecForActrTLe_ClimaCfgHvacReForRowSecForActrTLe_read/1 -> Terminator_65/1
  ClimaCfgHvacReForRowSecForActrTRi_ClimaCfgHvacReForRowSecForActrTRi_read/1 -> Terminator_66/1
  CmptmtClngActv_CmptmtClngActv_read/1 -> Terminator_67/1
  DsChrgClima_DsChrgClima_read/1 -> Terminator_68/1
  HMIClimaVentSetNgcc_HMIClimaVentSetNgcc_read/1 -> Terminator_69/1
  HvacProtdEnaWhenSetTempDiffWithHvah_HvacProtdEnaWhenSetTempDiffWithHvah_read/1 -> Terminator_70/1
  PwrCnsForHvacHeatrElecFrnt_PwrCnsForHvacHeatrElecFrnt_read/1 -> Terminator_71/1
  RemVentActvSts_RemVentActvSts_read/1 -> Terminator_72/1
  VentnForWinOpenGlbActv_VentnForWinOpenGlbActv_read/1 -> Terminator_73/1
  Ground_1/1 -> EnaFlapPosnLimForHvacProtd_EnaFlapPosnLimForHvacProtd_write/1
  Ground_2/1 -> HvacCtrlrReqFrntForRowFirstForActrTLe_HvacCtrlrReqFrntForRowFirstForActrTLe_write/1
  Ground_3/1 -> HvacCtrlrReqFrntForRowFirstForActrTRi_HvacCtrlrReqFrntForRowFirstForActrTRi_write/1
  Ground_4/1 -> HvacCtrlrReqFrntForRowSecForActrTLe_HvacCtrlrReqFrntForRowSecForActrTLe_write/1
  Ground_5/1 -> HvacCtrlrReqFrntForRowSecForActrTRi_HvacCtrlrReqFrntForRowSecForActrTRi_write/1
